simulink model "simulation_environment_thesis"  (R2024b)
settings: Solver=VariableStepAuto  StartTime=0.0  StopTime=3200  RelTol=1e-3
values from neighbouring files (symbols this model uses):
  A = [1, dt; 0, 1]    [estimate_and_control_fully_actuated_with_current.m]
  A1 = [1, 0, 0, 0, dt, 0 ... (first row only)    [estimate_and_control_fully_actuated_with_current.m]
  alpha = 10    [estimate_and_control_fully_actuated_with_current.m]
  alpha_xy_1 = 1    [estimate_and_control_fully_actuated_with_current.m]
  alpha_z_1 = 5    [estimate_and_control_fully_actuated_with_current.m]
  Az1 = [1, dt ... (first row only)    [estimate_and_control_fully_actuated_with_current.m]
  B = [dt; 0]    [estimate_and_control_fully_actuated_with_current.m]
  B1 = [dt, 0 ... (first row only)    [estimate_and_control_fully_actuated_with_current.m]
  Bz1 = [dt ... (first row only)    [estimate_and_control_fully_actuated_with_current.m]
  C1 = [1, 0, 0, 0, 0, 0 ... (first row only)    [estimate_and_control_fully_actuated_with_current.m]
  circumference_x = min_dist_from_ds*cos(theta)    [estimate_and_control_fully_actuated_with_current.m]
  circumference_y = min_dist_from_ds*sin(theta)    [estimate_and_control_fully_actuated_with_current.m]
  const_current = [-r; -s; 0; 0]    [estimate_and_control_fully_actuated_with_current.m]
  csi_psi_1 = 0.0003    [estimate_and_control_fully_actuated_with_current.m]
  csi_r_1 = 0.0003    [estimate_and_control_fully_actuated_with_current.m]
  csi_vcx_1 = 0.0003    [estimate_and_control_fully_actuated_with_current.m]
  csi_vcy_1 = 0.0003    [estimate_and_control_fully_actuated_with_current.m]
  csi_vcz_1 = 0.005    [estimate_and_control_fully_actuated_with_current.m]
  csi_x_1 = 0.003    [estimate_and_control_fully_actuated_with_current.m]
  csi_y_1 = 0.003    [estimate_and_control_fully_actuated_with_current.m]
  csi_z_1 = 0.005    [estimate_and_control_fully_actuated_with_current.m]
  Cz1 = [1, 0]    [estimate_and_control_fully_actuated_with_current.m]
  d = 1    [estimate_and_control_fully_actuated_with_current.m]
  delta_FOV = pi/7    (= 0.448798950513)    [estimate_and_control_fully_actuated_with_current.m]
  desired_speed = speed_0    [estimate_and_control_fully_actuated_with_current.m]
  dt = 1/Tsim    (= 0.005)    [estimate_and_control_fully_actuated_with_current.m]
  eta1 = deg2rad(0.5)    [estimate_and_control_fully_actuated_with_current.m]
  eta2 = deg2rad(0.5)    [estimate_and_control_fully_actuated_with_current.m]
  eta_psi_1 = 0.003    [estimate_and_control_fully_actuated_with_current.m]
  eta_x_1 = 0.03    [estimate_and_control_fully_actuated_with_current.m]
  eta_y_1 = 0.03    [estimate_and_control_fully_actuated_with_current.m]
  eta_z_1 = 0.005    [estimate_and_control_fully_actuated_with_current.m]
  G = dt*eye(2)    [estimate_and_control_fully_actuated_with_current.m]
  G1 = dt*eye(6)    [estimate_and_control_fully_actuated_with_current.m]
  Gz1 = dt*eye(2)    [estimate_and_control_fully_actuated_with_current.m]
  left_reference = L_x*[cos(NED_psi_ds); sin(NED_psi_ds); 0] + L_y_l*[-sin(NED_psi_ds); cos(NED_psi_ds); 0]    [estimate_and_control_fully_actuated_with_current.m]
  max_dist_from_ship = 60    [estimate_and_control_fully_actuated_with_current.m]
  min_dist_from_ds = 45    [estimate_and_control_fully_actuated_with_current.m]
  mu = -5    [estimate_and_control_fully_actuated_with_current.m]
  NED_psi_ds = -pi/2    (= -1.57079632679)    [estimate_and_control_fully_actuated_with_current.m]
  p_b1 = [-90; 90; 5]    [estimate_and_control_fully_actuated_with_current.m]
  position_t_0 = [0; 0; 0; 0]    [estimate_and_control_fully_actuated_with_current.m]
  psi_P = linspace(0,2*pi,360)'    [estimate_and_control_fully_actuated_with_current.m]
  Q = [csi1_kf^2, 0 ... (first row only)    [estimate_and_control_fully_actuated_with_current.m]
  Q1 = [csi_x_1_kf^2, 0, 0, 0, 0, 0 ... (first row only)    [estimate_and_control_fully_actuated_with_current.m]
  Qz1 = [csi_z_1_kf^2, 0 ... (first row only)    [estimate_and_control_fully_actuated_with_current.m]
  R = [eta1_kf^2, 0 ... (first row only)    [estimate_and_control_fully_actuated_with_current.m]
  R1 = [eta_x_1_kf^2, 0, 0 ... (first row only)    [estimate_and_control_fully_actuated_with_current.m]
  right_reference = L_x*[cos(NED_psi_ds); sin(NED_psi_ds); 0] + L_y_r*[sin(NED_psi_ds); -cos(NED_psi_ds); 0]    [estimate_and_control_fully_actuated_with_current.m]
  Rz1 = eta_z_1_kf^2    (= 1e-06)    [estimate_and_control_fully_actuated_with_current.m]
  speed_0 = [0.1, 0.1, 0, 0]'    [estimate_and_control_fully_actuated_with_current.m]
  tau_heave_sat = 30    [estimate_and_control_fully_actuated_with_current.m]
  tau_surge_sat = 10    [estimate_and_control_fully_actuated_with_current.m]
  tau_sway_sat = 30    [estimate_and_control_fully_actuated_with_current.m]
  tau_yaw_sat = 0.1    [estimate_and_control_fully_actuated_with_current.m]
  var_vm_x = 0.001    [estimate_and_control_fully_actuated_with_current.m]
  var_vm_y = 0.001    [estimate_and_control_fully_actuated_with_current.m]
  var_vm_z = 0.001    [estimate_and_control_fully_actuated_with_current.m]
undefined symbols (not defined in the file or in its folder): A2, alpha2, B2, csi1_2, csi2_2, csi3_2, csi4_2, csi5_2, csi6_2, csi7_2, csi8_2, eta1_2, eta2_2, eta3_2, eta4_2, eta5_2, eta6_2, G2, Q2, R2
libraries: simulink x60, simulink_extras x5
(part 1 of 16)
settings Model: Creator=ambra  LastModifiedBy=ambra

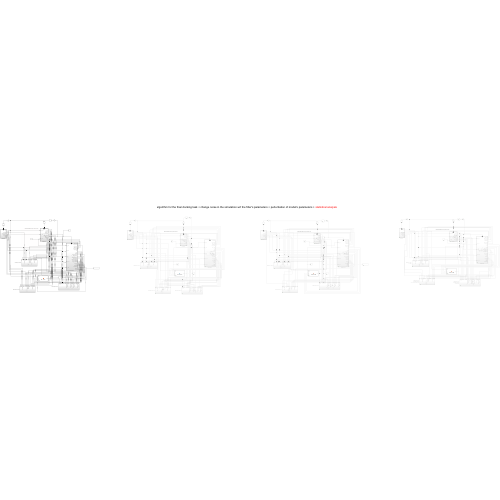
[thumbnail: Simulink's stored preview of the top-level diagram]
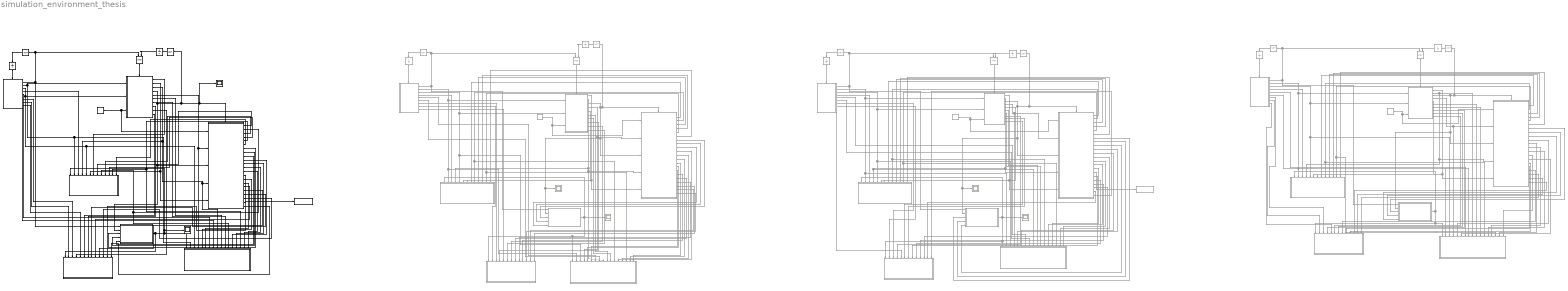
== system simulation_environment_thesis ==
  note: before transformation from ship coord to ds coord
  note: algorithm for the final docking task + change noise in the simulation wrt the filter's parameters + perturbation of model's parameters + statistical analysis
  note: before transformation from ship coord to ds coord
  note: before transformation from ship coord to ds coord
  note: before transformation from ship coord to ds coord
  AND21 : Logic  Commented=on  IconShape=distinctive  AllPortsSameDT=off  OutDataTypeStr=boolean
  AND22 : Logic  Commented=on  IconShape=distinctive  AllPortsSameDT=off  OutDataTypeStr=boolean
  AND23 : Logic  Commented=on  IconShape=distinctive  AllPortsSameDT=off  OutDataTypeStr=boolean
  AND24 : Logic  IconShape=distinctive  AllPortsSameDT=off  OutDataTypeStr=boolean
  Constant30 : Constant  Value=p_b1
  Constant31 : Constant  Commented=on  Value=p_b1
  Constant32 : Constant  Commented=on  Value=p_b1
  Constant33 : Constant  Commented=on  Value=p_b1
  MATLAB Function27 : MATLAB Function  TreatAsAtomicUnit=on
  MATLAB Function28 : MATLAB Function  Commented=on  TreatAsAtomicUnit=on
  MATLAB Function29 : MATLAB Function  Commented=on  TreatAsAtomicUnit=on
  MATLAB Function30 : MATLAB Function  Commented=on  TreatAsAtomicUnit=on
  NOT43 : Logic  Operator=NOT  IconShape=distinctive  AllPortsSameDT=off  OutDataTypeStr=boolean
  NOT44 : Logic  Operator=NOT  IconShape=distinctive  AllPortsSameDT=off  OutDataTypeStr=boolean
  NOT{45..50} (6 alike) : Logic  Commented=on  Operator=NOT  IconShape=distinctive  AllPortsSameDT=off  OutDataTypeStr=boolean
  "PHASE 1  " : Subsystem  Commented=on
  PHASE 1.21 : Subsystem  Commented=on
  PHASE 1.22 : Subsystem  Commented=on
  PHASE 1.24 : Subsystem
  "PHASE 2  " : Subsystem  Commented=on
  PHASE 2.21 : Subsystem  Commented=on
  PHASE 2.22 : Subsystem  Commented=on
  PHASE 2.24 : Subsystem
  "PHASE 3&4  " : Subsystem  Commented=on
  PHASE 3&4.21 : Subsystem  Commented=on
  PHASE 3&4.22 : Subsystem  Commented=on
  PHASE 3&4.24 : Subsystem
  Scope50 : Scope
  Scope51 : Scope
  Scope{53..54,56..57} (4 alike) : Scope  Commented=on
  To Workspace15 : ToWorkspace  VariableName=yaw_rel_error1  SampleTime=-1
  To Workspace16 : ToWorkspace  Commented=on  VariableName=yaw_rel_error  SampleTime=-1
  Unit Delay43 : UnitDelay  InitialCondition=1  SampleTime=-1  HasFrameUpgradeWarning=on
  Unit Delay44 : UnitDelay  InitialCondition=1  SampleTime=-1  HasFrameUpgradeWarning=on
  Unit Delay{45..50} (6 alike) : UnitDelay  Commented=on  InitialCondition=1  SampleTime=-1  HasFrameUpgradeWarning=on
  toWorkspace blocks for plotting : Subsystem  Commented=on
  toWorkspace blocks for statistical analysis on the error wrt the references : Subsystem  Commented=on
  "toWorkspace blocks for statistical analysis on the estimate  " : Subsystem  Commented=on
  toWorkspace blocks 21 : Subsystem  Commented=on
  toWorkspace blocks 22 : Subsystem  Commented=on
  toWorkspace blocks 24 : Subsystem
  toWorkspace blocks 25 : Subsystem
  toWorkspace blocks 26 : Subsystem  Commented=on
  toWorkspace blocks 27 : Subsystem  Commented=on
  toWorkspace blocks 29 : Subsystem
  toWorkspace blocks 30 : Subsystem  Commented=on
  toWorkspace blocks 31 : Subsystem  Commented=on
  wires:
  PHASE 1.21/3(p) -> toWorkspace blocks 26/3(p1), toWorkspace blocks 21/5(p1), PHASE 2.21/2(ship_p_auv)
  PHASE 1.21/1(stop_flag) -> NOT45/1, AND21/1, MATLAB Function28/1(stop1)
  Constant32/1 -> PHASE 3&4.22/1(ship_p_ds), PHASE 2.22/3(ship_p_ds)
  Constant31/1 -> PHASE 3&4.21/1(ship_p_ds), PHASE 2.21/3(ship_p_ds)
  PHASE 3&4.22/18(yaw_error) -> toWorkspace blocks 31/9(psi3)
  PHASE 2.24/2(s_p_auv) -> PHASE 3&4.24/5(ship_p_auv), toWorkspace blocks 24/6(p2)
  PHASE 3&4.21/16("z_err ") -> toWorkspace blocks 30/10(p3)
  PHASE 1.24/5(state_est_plane) -> toWorkspace blocks 25/7(state_est_plane_1)
  "PHASE 2  "/8(angle) -> toWorkspace blocks for statistical analysis on the error wrt the references/4(psi2)
  PHASE 1.24/6(state_est_z) -> toWorkspace blocks 25/8(state_est_z_1)
  "PHASE 2  "/9(error speed) -> toWorkspace blocks for statistical analysis on the error wrt the references/7(speed body2)
  "PHASE 3&4  "/13(state_est_plane3) -> "toWorkspace blocks for statistical analysis on the estimate  "/13(state_est_plane_3)
  "PHASE 3&4  "/11(state_est_plane4) -> "toWorkspace blocks for statistical analysis on the estimate  "/16(state_est_plane_4)
  Unit Delay48/1 -> AND22/2
  PHASE 3&4.24/21(angle 4) -> toWorkspace blocks 29/12(psi4)
  "PHASE 3&4  "/8(out1) -> MATLAB Function30/4(out1)
  PHASE 3&4.24/19(error speed) -> toWorkspace blocks 29/8(speed body3)
  PHASE 3&4.24/16(ds_p_auv_phase4) -> toWorkspace blocks 25/6(p4)
  "PHASE 2  "/10(z_ err) -> toWorkspace blocks for statistical analysis on the error wrt the references/6(p2)
  PHASE 3&4.21/4(speed body1) -> toWorkspace blocks 21/11(speed_body4)
  "PHASE 3&4  "/12(state_est_z3) -> "toWorkspace blocks for statistical analysis on the estimate  "/14(state_est_z_3)
  PHASE 3&4.24/6(ship_p_auv2) -> toWorkspace blocks 24/13(p4)
  PHASE 3&4.22/4(speed body1) -> toWorkspace blocks 22/11(speed_body4)
  "PHASE 2  "/4(ds_p_auv) -> "toWorkspace blocks for statistical analysis on the estimate  "/4(p2), "PHASE 3&4  "/2(ds_p_auv)
  "PHASE 3&4  "/17(yaw_error) -> toWorkspace blocks for statistical analysis on the error wrt the references/9(psi3)
  "PHASE 1  "/2(psi) -> "toWorkspace blocks for statistical analysis on the estimate  "/2(psi1), toWorkspace blocks for plotting/2(psi1), "PHASE 2  "/1(ship_psi_auv)
  "PHASE 1  "/3(p) -> "toWorkspace blocks for statistical analysis on the estimate  "/3(p1), toWorkspace blocks for plotting/5(p1), "PHASE 2  "/2(ship_p_auv)
  PHASE 3&4.24/18("z_err ") -> toWorkspace blocks 29/10(p3)
  Unit Delay43/1 -> PHASE 1.24/Enable
  PHASE 2.21/6(state_est_plane) -> toWorkspace blocks 26/10(state_est_plane_2)
  PHASE 3&4.21/17(error speed) -> toWorkspace blocks 30/8(speed body3)
  PHASE 2.24/6(state_est_plane) -> toWorkspace blocks 25/10(state_est_plane_2)
  NOT44/1 -> Unit Delay44/1
  "PHASE 3&4  "/10(ds_p_auv_3) -> "toWorkspace blocks for statistical analysis on the estimate  "/5(p3)
  PHASE 1.22/8(angle) -> toWorkspace blocks 31/2(psi1)
  PHASE 3&4.21/10(state_est_plane_3) -> toWorkspace blocks 26/13(state_est_plane_3)
  PHASE 3&4.21/6(ship_p_auv2) -> toWorkspace blocks 21/13(p4)
  PHASE 2.22/9(error speed) -> toWorkspace blocks 31/7(speed body2)
  "PHASE 1  "/9(z_ err) -> toWorkspace blocks for statistical analysis on the error wrt the references/5(p1)
  Unit Delay49/1 -> AND23/2
  Unit Delay44/1 -> AND24/2
  "PHASE 1  "/6(state_est_z) -> "toWorkspace blocks for statistical analysis on the estimate  "/8(state_est_z_1)
  "PHASE 3&4  "/7(out) -> MATLAB Function30/3(out)
  PHASE 3&4.22/12(state_est_plane4) -> toWorkspace blocks 27/16(state_est_plane_4)
  "PHASE 3&4  "/19(z_ err 4) -> toWorkspace blocks for statistical analysis on the error wrt the references/13(p4)
  PHASE 1.21/9(z_ err) -> toWorkspace blocks 30/5(p1)
  PHASE 3&4.24/15(ds_p_auv_phase3) -> toWorkspace blocks 25/5(p3)
  "PHASE 2  "/2(s_psi_auv) -> toWorkspace blocks for plotting/4(psi2), "PHASE 3&4  "/4(ship_psi_auv), "toWorkspace blocks for statistical analysis on the estimate  "/9(psi2)
  PHASE 1.22/6(state_z) -> toWorkspace blocks 27/8(state_est_z_1)
  PHASE 3&4.22/21(angle 4) -> toWorkspace blocks 31/12(psi4)
  PHASE 1.21/6(state_est_z) -> toWorkspace blocks 26/8(state_est_z_1)
  PHASE 1.24/2(psi) -> PHASE 2.24/1(ship_psi_auv), toWorkspace blocks 25/2(psi1), toWorkspace blocks 24/2(psi1)
  PHASE 3&4.22/10(ds_p_auv_3) -> toWorkspace blocks 27/5(p3)
  AND24/1 -> PHASE 2.24/Enable
  PHASE 3&4.22/20(error speed 4) -> toWorkspace blocks 31/11(speed_body4)
  PHASE 1.21/8(angle) -> toWorkspace blocks 30/2(psi1)
  PHASE 3&4.22/1(speed body) -> toWorkspace blocks 22/8(speed body3)
  PHASE 3&4.24/22(z err 4) -> toWorkspace blocks 29/13(p4)
  PHASE 3&4.24/1(speed body) -> toWorkspace blocks 24/8(speed body3)
  "PHASE 1  "/5(state_est_plane) -> "toWorkspace blocks for statistical analysis on the estimate  "/7(state_est_plane_1)
  "PHASE 1  "/7(speed error) -> toWorkspace blocks for statistical analysis on the error wrt the references/3(body_speed_1)
  PHASE 3&4.24/5(ship_psi_auv2) -> toWorkspace blocks 24/12(psi4), toWorkspace blocks 25/15(psi4)
  Unit Delay46/1 -> AND21/2
  MATLAB Function27/1(state) -> toWorkspace blocks 24/1(state), Scope50/1, toWorkspace blocks 25/1(state), toWorkspace blocks 29/1(state)
  PHASE 3&4.22/22("z_ err 4 ") -> toWorkspace blocks 31/13(p4)
  PHASE 3&4.22/8(out1) -> MATLAB Function29/4(out1)
  PHASE 3&4.21/20(angle 4) -> toWorkspace blocks 30/12(psi4)
  PHASE 2.22/10(z_ err) -> toWorkspace blocks 31/6(p2)
  PHASE 1.21/7(error speed) -> toWorkspace blocks 30/3(body_speed_1)
  "PHASE 2  "/3(stop_flag_2) -> "PHASE 3&4  "/3(stop_flag), MATLAB Function30/2(stop2), "PHASE 3&4  "/Enable, NOT49/1
  "PHASE 1  "/1(stop_flag) -> AND23/1, NOT50/1, MATLAB Function30/1(stop1)
  PHASE 2.22/2(s_psi_auv) -> PHASE 3&4.22/4(ship_psi_auv), toWorkspace blocks 27/9(psi2), toWorkspace blocks 22/4(psi2)
  PHASE 3&4.21/8(out1) -> MATLAB Function28/4(out1)
  PHASE 3&4.24/3(ship_p_auv1) -> toWorkspace blocks 24/10(p3)
  PHASE 3&4.22/16(yaw_rel_err) -> To Workspace16/1
  PHASE 1.22/1(stop_flag) -> NOT47/1, AND22/1, MATLAB Function29/1(stop1)
  PHASE 3&4.22/19(z_ err) -> toWorkspace blocks 31/10(p3)
  PHASE 1.21/4(body speed) -> toWorkspace blocks 21/3(body_speed_1)
  PHASE 2.21/8(angle) -> toWorkspace blocks 30/4(psi2)
  MATLAB Function29/1(state) -> toWorkspace blocks 31/1(state), toWorkspace blocks 27/1(state), Scope56/1, toWorkspace blocks 22/1(state)
  PHASE 3&4.24/10(state_est_plane_3) -> toWorkspace blocks 25/13(state_est_plane_3)
  PHASE 2.24/5(ds_p_auv) -> toWorkspace blocks 25/4(p2), PHASE 3&4.24/2(ds_p_auv)
  PHASE 2.21/9(error speed) -> toWorkspace blocks 30/7(speed body2)
  PHASE 1.24/7(error speed) -> toWorkspace blocks 29/3(body_speed_1)
  PHASE 3&4.24/7(out) -> MATLAB Function27/3(out)
  PHASE 3&4.21/14(ds_p_auv_3) -> toWorkspace blocks 26/5(p3)
  PHASE 2.22/3(stop_flag_2) -> PHASE 3&4.22/3(stop_flag), Scope57/1, MATLAB Function29/2(stop2), NOT48/1, PHASE 3&4.22/Enable
  PHASE 1.22/7(error speed) -> toWorkspace blocks 31/3(body_speed_1)
  PHASE 3&4.24/14(state_est_yaw_rel_4) -> To Workspace15/1
  PHASE 3&4.22/17(error speed) -> toWorkspace blocks 31/8(speed body3)
  PHASE 1.22/3(p) -> toWorkspace blocks 27/3(p1), toWorkspace blocks 22/5(p1), PHASE 2.22/2(ship_p_auv)
  PHASE 3&4.22/11(ds_p_auv4) -> toWorkspace blocks 27/6(p4)
  NOT45/1 -> Unit Delay45/1
  PHASE 2.21/1(s_p_auv) -> PHASE 3&4.21/5(ship_p_auv), toWorkspace blocks 21/6(p2)
  PHASE 2.22/6(state_est_plane) -> toWorkspace blocks 27/10(state_est_plane_2)
  PHASE 3&4.21/3(ship_p_auv1) -> toWorkspace blocks 21/10(p3)
  PHASE 2.21/7(state_est_z) -> toWorkspace blocks 26/11(state_est_z_2)
  PHASE 3&4.24/17(yaw_error) -> toWorkspace blocks 29/9(psi3)
  PHASE 2.24/7(state_est_z) -> toWorkspace blocks 25/11(state_est_z_2)
  Unit Delay50/1 -> "PHASE 1  "/Enable
  PHASE 3&4.22/6(ship_p_auv2) -> toWorkspace blocks 22/13(p4)
  PHASE 1.21/5(state_est_plane) -> toWorkspace blocks 26/7(state_est_plane_1)
  PHASE 3&4.24/4(speed body1) -> toWorkspace blocks 24/11(speed_body4)
  "PHASE 3&4  "/18(error speed) -> toWorkspace blocks for statistical analysis on the error wrt the references/8(speed body3)
  PHASE 2.21/2(s_psi_auv) -> PHASE 3&4.21/4(ship_psi_auv), toWorkspace blocks 26/9(psi2), toWorkspace blocks 21/4(psi2)
  PHASE 3&4.24/20(error speed 4) -> toWorkspace blocks 29/11(speed_body4)
  PHASE 2.22/4(ds_p_auv) -> toWorkspace blocks 27/4(p2), PHASE 3&4.22/2(ds_p_auv)
  PHASE 3&4.22/9(docked) -> MATLAB Function29/5(docked)
  PHASE 3&4.21/11(state_est_z_3) -> toWorkspace blocks 26/14(state_est_z_3)
  PHASE 2.24/3(s_psi_auv) -> toWorkspace blocks 25/9(psi2), PHASE 3&4.24/4(ship_psi_auv), toWorkspace blocks 24/4(psi2)
  AND22/1 -> PHASE 2.22/Enable
  PHASE 1.24/1(stop_flag) -> AND24/1, NOT43/1, MATLAB Function27/1(stop1)
  PHASE 2.24/10("z_err ") -> toWorkspace blocks 29/6(p2)
  PHASE 1.22/4(body speed) -> toWorkspace blocks 22/3(body_speed_1)
  PHASE 3&4.22/15(state_est_z3) -> toWorkspace blocks 27/14(state_est_z_3)
  "PHASE 1  "/4(body speed) -> toWorkspace blocks for plotting/3(body_speed_1)
  PHASE 1.24/8(angle) -> toWorkspace blocks 29/2(psi1)
  PHASE 2.24/9(angle) -> toWorkspace blocks 29/4(psi2)
  MATLAB Function30/1(state) -> toWorkspace blocks for statistical analysis on the error wrt the references/1(state), "toWorkspace blocks for statistical analysis on the estimate  "/1(state), toWorkspace blocks for plotting/1(state)
  "PHASE 3&4  "/15(ds_p_auv4) -> "toWorkspace blocks for statistical analysis on the estimate  "/6(p4)
  PHASE 3&4.24/2(ship_psi_auv1) -> toWorkspace blocks 24/9(psi3), toWorkspace blocks 25/12(psi3)
  "PHASE 3&4  "/5(ship_psi_auv2) -> "toWorkspace blocks for statistical analysis on the estimate  "/15(psi4), toWorkspace blocks for plotting/12(psi4)
  PHASE 1.22/9("z_err ") -> toWorkspace blocks 31/5(p1)
  "PHASE 3&4  "/3(ship_p_auv1) -> toWorkspace blocks for plotting/10(p3)
  PHASE 1.24/4(body speed) -> toWorkspace blocks 24/3(body_speed_1)
  "PHASE 2  "/6(state_est_plane) -> "toWorkspace blocks for statistical analysis on the estimate  "/10(state_est_plane_2)
  PHASE 1.22/2(psi) -> toWorkspace blocks 27/2(psi1), toWorkspace blocks 22/2(psi1), PHASE 2.22/1(ship_psi_auv)
  PHASE 2.22/8(angle) -> toWorkspace blocks 31/4(psi2)
  PHASE 3&4.22/13(state_est_z4) -> toWorkspace blocks 27/17(state_est_z_4)
  PHASE 3&4.21/2(ship_psi_auv1) -> toWorkspace blocks 26/12(psi3), toWorkspace blocks 21/9(psi3)
  PHASE 2.22/5(body speed) -> toWorkspace blocks 22/7(speed body2)
  PHASE 1.22/5(state_plane) -> toWorkspace blocks 27/7(state_est_plane_1)
  PHASE 3&4.22/2(ship_psi_auv1) -> toWorkspace blocks 27/12(psi3), toWorkspace blocks 22/9(psi3)
  PHASE 3&4.22/7(out) -> MATLAB Function29/3(out)
  NOT43/1 -> Unit Delay43/1
  "PHASE 3&4  "/16(z_ err) -> toWorkspace blocks for statistical analysis on the error wrt the references/10(p3)
  PHASE 3&4.21/9(docked) -> MATLAB Function28/5(docked)
  PHASE 3&4.21/5(ship_psi_auv2) -> toWorkspace blocks 26/15(psi4), toWorkspace blocks 21/12(psi4)
  "PHASE 3&4  "/21(error speed 4) -> toWorkspace blocks for statistical analysis on the error wrt the references/11(speed_body4)
  PHASE 3&4.21/12(state_est_plane_4) -> toWorkspace blocks 26/16(state_est_plane_4)
  PHASE 1.24/3(p) -> toWorkspace blocks 25/3(p1), toWorkspace blocks 24/5(p1), PHASE 2.24/2(ship_p_auv)
  PHASE 3&4.24/12(state_est_plane_4) -> toWorkspace blocks 25/16(state_est_plane_4)
  PHASE 3&4.21/21(error speed 4) -> toWorkspace blocks 30/11(speed_body4)
  PHASE 3&4.21/18(yaw_error) -> toWorkspace blocks 30/9(psi3)
  AND23/1 -> "PHASE 2  "/Enable
  MATLAB Function28/1(state) -> toWorkspace blocks 30/1(state), toWorkspace blocks 26/1(state), toWorkspace blocks 21/1(state), Scope53/1
  PHASE 3&4.21/1(speed body) -> toWorkspace blocks 21/8(speed body3)
  "PHASE 3&4  "/6(ship_p_auv2) -> toWorkspace blocks for plotting/13(p4)
  "PHASE 1  "/8(yaw_error) -> toWorkspace blocks for statistical analysis on the error wrt the references/2(psi1)
  "PHASE 2  "/5(body speed) -> toWorkspace blocks for plotting/7(speed body2)
  "PHASE 3&4  "/1(speed body) -> toWorkspace blocks for plotting/8(speed body3)
  PHASE 2.21/4(ds_p_auv) -> toWorkspace blocks 26/4(p2), PHASE 3&4.21/2(ds_p_auv)
  Constant30/1 -> PHASE 3&4.24/1(ship_p_ds), PHASE 2.24/3(ship_p_ds)
  Unit Delay45/1 -> PHASE 1.21/Enable
  Unit Delay47/1 -> PHASE 1.22/Enable
  PHASE 2.22/7(state_est_z) -> toWorkspace blocks 27/11(state_est_z_2)
  "PHASE 3&4  "/9(docked) -> MATLAB Function30/5(docked)
  PHASE 3&4.24/8(out1) -> MATLAB Function27/4(out1)
  PHASE 3&4.24/13(state_est_z_4) -> toWorkspace blocks 25/17(state_est_z_4)
  "PHASE 3&4  "/14(state_est_z4) -> "toWorkspace blocks for statistical analysis on the estimate  "/17(state_est_z_4)
  PHASE 3&4.21/7(out) -> MATLAB Function28/3(out)
  NOT50/1 -> Unit Delay50/1
  PHASE 3&4.21/13(state_est_z_4) -> toWorkspace blocks 26/17(state_est_z_4)
  PHASE 3&4.22/5(ship_psi_auv2) -> toWorkspace blocks 27/15(psi4), toWorkspace blocks 22/12(psi4)
  PHASE 1.21/2(psi) -> toWorkspace blocks 26/2(psi1), toWorkspace blocks 21/2(psi1), PHASE 2.21/1(ship_psi_auv)
  NOT49/1 -> Unit Delay49/1
  PHASE 3&4.22/14(state_est_plane3) -> toWorkspace blocks 27/13(state_est_plane_3)
  Constant33/1 -> "PHASE 3&4  "/1(ship_p_ds), "PHASE 2  "/3(ship_p_ds)
  PHASE 3&4.24/9(docked) -> MATLAB Function27/5(docked)
  PHASE 2.21/5(body speed) -> toWorkspace blocks 21/7(speed body2)
  PHASE 2.21/10(z_ err) -> toWorkspace blocks 30/6(p2)
  "PHASE 3&4  "/2(ship_psi_auv1) -> "toWorkspace blocks for statistical analysis on the estimate  "/12(psi3), toWorkspace blocks for plotting/9(psi3)
  "PHASE 2  "/1(s_p_auv) -> "PHASE 3&4  "/5(ship_p_auv), toWorkspace blocks for plotting/6(p2)
  PHASE 3&4.21/15(ds_p_auv_4) -> toWorkspace blocks 26/6(p4)
  PHASE 2.22/1(s_p_auv) -> PHASE 3&4.22/5(ship_p_auv), toWorkspace blocks 22/6(p2)
  PHASE 2.24/8(error speed) -> toWorkspace blocks 29/7(speed body2)
  PHASE 2.21/3(stop_flag_2) -> PHASE 3&4.21/3(stop_flag), Scope54/1, MATLAB Function28/2(stop2), PHASE 3&4.21/Enable, NOT46/1
  PHASE 2.24/1(speed body) -> toWorkspace blocks 24/7(speed body2)
  "PHASE 2  "/7(state_est_z) -> "toWorkspace blocks for statistical analysis on the estimate  "/11(state_est_z_2)
  PHASE 3&4.22/3(ship_p_auv1) -> toWorkspace blocks 22/10(p3)
  NOT46/1 -> Unit Delay46/1
  "PHASE 3&4  "/20(angle 4) -> toWorkspace blocks for statistical analysis on the error wrt the references/12(psi4)
  NOT47/1 -> Unit Delay47/1
  PHASE 3&4.21/19(z_err 4) -> toWorkspace blocks 30/13(p4)
  AND21/1 -> PHASE 2.21/Enable
  PHASE 2.24/4(stop_flag_2) -> NOT44/1, PHASE 3&4.24/Enable, Scope51/1, MATLAB Function27/2(stop2), PHASE 3&4.24/3(stop_flag)
  PHASE 3&4.24/11(state_est_z_3) -> toWorkspace blocks 25/14(state_est_z_3)
  "PHASE 3&4  "/4(speed body1) -> toWorkspace blocks for plotting/11(speed_body4)
  PHASE 1.24/9("z_err ") -> toWorkspace blocks 29/5(p1)
  NOT48/1 -> Unit Delay48/1

== system simulation_environment_thesis/PHASE 1   ==
  note: PHASE 1
  Enable : EnablePort
  CONTROLLER1 : Subsystem
  Constant2 : Constant  Value=p_b1(3)
  Data Store Write : DataStoreWrite  DataStoreName=x_hat_12_xy_4  IndexOptions=Index vector (dialog)  Indices=1  OutputSizes=1
  Data Store Write1 : DataStoreWrite  DataStoreName=x_hat_12_z_4  IndexOptions=Index vector (dialog)  Indices=1  OutputSizes=1
  Data Store Write2 : DataStoreWrite  DataStoreName=P0_12_xy_4  IndexOptions=Index vector (dialog)  Indices=1  OutputSizes=1
  Data Store Write3 : DataStoreWrite  DataStoreName=P0_12_z_4  IndexOptions=Index vector (dialog)  Indices=1  OutputSizes=1
  REFERENCE GENERATOR : Subsystem
  Scope : Scope
  Selector : Selector  InputPortWidth=4  IndexOptions=Index vector (dialog)  Indices=[1 2]  OutputSizes=1
  Sum : Sum  Inputs=|+-
  Sum1 : Sum  Inputs=|-+
  TAU : Scope
  dynamic + kinematic models1 : Subsystem
  v error (robot frame) : Scope
  "x,y,psi,r,vc estimate, XY PLANE from x,y,psi" : Subsystem
  "z, v_c_z estimate from DEPTH measurement" : Subsystem
  z_err : Scope
  stop_flag : Outport
  psi : Outport  Port=2
  p : Outport  Port=3
  body speed : Outport  Port=4
  state_est_plane : Outport  Port=5
  state_est_z : Outport  Port=6
  speed error : Outport  Port=7
  yaw_error : Outport  Port=8
  z_ err : Outport  Port=9
  wires:
  dynamic + kinematic models1/2(v_tot inert) -> Selector/1
  CONTROLLER1/1(tau) -> dynamic + kinematic models1/1(tau), TAU/1
  dynamic + kinematic models1/3(psi) -> "x,y,psi,r,vc estimate, XY PLANE from x,y,psi"/3(psi), psi/1, dynamic + kinematic models1/3(yaw prev)
  Selector/1 -> "x,y,psi,r,vc estimate, XY PLANE from x,y,psi"/2(inert_vel)
  dynamic + kinematic models1/1(error speed) -> CONTROLLER1/1(v_err), v error (robot frame)/1, speed error/1
  dynamic + kinematic models1/4(p) -> "z, v_c_z estimate from DEPTH measurement"/1(p), "x,y,psi,r,vc estimate, XY PLANE from x,y,psi"/1(p), p/1
  "z, v_c_z estimate from DEPTH measurement"/1(z_est) -> Sum/2
  Constant2/1 -> Sum/1
  Sum/1 -> z_ err/1, z_err/1, CONTROLLER1/4(z_err)
  "x,y,psi,r,vc estimate, XY PLANE from x,y,psi"/2(psi_est) -> Sum1/1
  Sum1/1 -> Scope/1, CONTROLLER1/3(yaw_err), yaw_error/1
  dynamic + kinematic models1/5(body speed) -> body speed/1
  "x,y,psi,r,vc estimate, XY PLANE from x,y,psi"/4(state_est) -> Data Store Write/1
  "z, v_c_z estimate from DEPTH measurement"/2(state_est) -> Data Store Write1/1
  "x,y,psi,r,vc estimate, XY PLANE from x,y,psi"/5(P_knext_knext) -> Data Store Write2/1
  "z, v_c_z estimate from DEPTH measurement"/3(P_k_k) -> Data Store Write3/1
  "x,y,psi,r,vc estimate, XY PLANE from x,y,psi"/6(state_est_plane) -> state_est_plane/1
  "z, v_c_z estimate from DEPTH measurement"/4(state_est_z) -> state_est_z/1
  REFERENCE GENERATOR/1(speed) -> dynamic + kinematic models1/2(ref speed), CONTROLLER1/2(speed_0)
  "x,y,psi,r,vc estimate, XY PLANE from x,y,psi"/1(xy_est) -> REFERENCE GENERATOR/1(p)
  "x,y,psi,r,vc estimate, XY PLANE from x,y,psi"/3(v_c_xy) -> REFERENCE GENERATOR/2(v_c)
  REFERENCE GENERATOR/2(yaw_dock) -> Sum1/2
  REFERENCE GENERATOR/3(stop_flag) -> stop_flag/1

== system simulation_environment_thesis/PHASE 1.21 ==
  note: USO I VALORI NOMINALI, NON HO NESSUN FEEDBACK SULLE VELOCITà EFFETTIVE NEL BODY FRAME
  note: ship reference frame
  note: PHASE 1:
  Enable : EnablePort
  CONTROLLER1 : Subsystem
  Constant : Constant  Value=p_b1
  Constant1 : Constant  Value=max_dist_from_ship
  Constant2 : Constant  Value=p_b1(3)
  Constant5 : Constant  Value=desired_speed
  Data Store Write : DataStoreWrite  DataStoreName=x_hat_12_xy_2  IndexOptions=Index vector (dialog)  Indices=1  OutputSizes=1
  Data Store Write1 : DataStoreWrite  DataStoreName=x_hat_12_z_2  IndexOptions=Index vector (dialog)  Indices=1  OutputSizes=1
  Data Store Write2 : DataStoreWrite  DataStoreName=P0_12_xy_2  IndexOptions=Index vector (dialog)  Indices=1  OutputSizes=1
  Data Store Write3 : DataStoreWrite  DataStoreName=P0_12_z_2  IndexOptions=Index vector (dialog)  Indices=1  OutputSizes=1
  MATLAB Function : MATLAB Function  TreatAsAtomicUnit=on
  MATLAB Function7 : MATLAB Function  TreatAsAtomicUnit=on
  Memory : Memory
  Scope : Scope
  Selector : Selector  InputPortWidth=4  IndexOptions=Index vector (dialog)  Indices=[1 2]  OutputSizes=1
  Sum : Sum  Inputs=|+-
  Sum1 : Sum  Inputs=|-+
  TAU1 : Scope
  dynamic + kinematic models3 : Subsystem
  v error (robot frame)4 : Scope
  "x,y,psi,r,vc estimate, XY PLANE from x,y,psi" : Subsystem
  "z, v_c_z estimate from DEPTH measurement" : Subsystem
  z_err : Scope
  stop_flag : Outport
  psi : Outport  Port=2
  p : Outport  Port=3
  body speed : Outport  Port=4
  state_est_plane : Outport  Port=5
  state_est_z : Outport  Port=6
  error speed : Outport  Port=7
  angle : Outport  Port=8
  z_ err : Outport  Port=9
  wires:
  dynamic + kinematic models3/2(v_tot inert) -> Selector/1
  CONTROLLER1/1(tau) -> dynamic + kinematic models3/1(tau), TAU1/1
  dynamic + kinematic models3/3(psi) -> dynamic + kinematic models3/3(yaw prev), "x,y,psi,r,vc estimate, XY PLANE from x,y,psi"/3(psi), psi/1
  Constant5/1 -> CONTROLLER1/2(speed_0), MATLAB Function7/5(speed), dynamic + kinematic models3/2(ref speed)
  Selector/1 -> "x,y,psi,r,vc estimate, XY PLANE from x,y,psi"/2(inert_vel)
  dynamic + kinematic models3/1(error speed) -> CONTROLLER1/1(v_err), error speed/1, v error (robot frame)4/1
  dynamic + kinematic models3/4(p) -> "z, v_c_z estimate from DEPTH measurement"/1(p), p/1, "x,y,psi,r,vc estimate, XY PLANE from x,y,psi"/1(p)
  "z, v_c_z estimate from DEPTH measurement"/1(z_est) -> Sum/2
  Constant2/1 -> Sum/1
  Sum/1 -> z_ err/1, z_err/1, CONTROLLER1/4(z_err)
  "x,y,psi,r,vc estimate, XY PLANE from x,y,psi"/1(xy_est) -> MATLAB Function7/3(p)
  "x,y,psi,r,vc estimate, XY PLANE from x,y,psi"/3(v_c_xy) -> MATLAB Function7/2(v_c)
  Constant/1 -> MATLAB Function7/4(beacon)
  Constant1/1 -> MATLAB Function7/6(max_distance_from_ship)
  Memory/1 -> MATLAB Function7/1("stop_flag ")
  MATLAB Function7/2(stop_flag) -> Memory/1, stop_flag/1
  "x,y,psi,r,vc estimate, XY PLANE from x,y,psi"/2(psi_est) -> Sum1/1
  MATLAB Function7/1(yaw_desired) -> Sum1/2
  Sum1/1 -> MATLAB Function/1("angle ")
  MATLAB Function/1(angle) -> angle/1, CONTROLLER1/3(yaw_err), Scope/1
  dynamic + kinematic models3/5(body speed) -> body speed/1
  "x,y,psi,r,vc estimate, XY PLANE from x,y,psi"/4(state_est) -> Data Store Write/1
  "z, v_c_z estimate from DEPTH measurement"/2(state_est) -> Data Store Write1/1
  "x,y,psi,r,vc estimate, XY PLANE from x,y,psi"/5(P_knext_knext) -> Data Store Write2/1
  "z, v_c_z estimate from DEPTH measurement"/3(P_k_k) -> Data Store Write3/1
  "x,y,psi,r,vc estimate, XY PLANE from x,y,psi"/6(state_est_plane) -> state_est_plane/1
  "z, v_c_z estimate from DEPTH measurement"/4(state_est_z) -> state_est_z/1

== system simulation_environment_thesis/PHASE 1.22 ==
  note: USO I VALORI NOMINALI, NON HO NESSUN FEEDBACK SULLE VELOCITà EFFETTIVE NEL BODY FRAME
  note: ship reference frame
  note: PHASE 1:
  Enable : EnablePort
  CONTROLLER1 : Subsystem
  Constant : Constant  Value=p_b1
  Constant1 : Constant  Value=max_dist_from_ship
  Constant2 : Constant  Value=p_b1(3)
  Constant5 : Constant  Value=desired_speed
  Data Store Write : DataStoreWrite  DataStoreName=x_hat_12_xy_3  IndexOptions=Index vector (dialog)  Indices=1  OutputSizes=1
  Data Store Write1 : DataStoreWrite  DataStoreName=x_hat_12_z_3  IndexOptions=Index vector (dialog)  Indices=1  OutputSizes=1
  Data Store Write2 : DataStoreWrite  DataStoreName=P0_12_xy_3  IndexOptions=Index vector (dialog)  Indices=1  OutputSizes=1
  Data Store Write3 : DataStoreWrite  DataStoreName=P0_12_z_3  IndexOptions=Index vector (dialog)  Indices=1  OutputSizes=1
  MATLAB Function : MATLAB Function  TreatAsAtomicUnit=on
  MATLAB Function7 : MATLAB Function  TreatAsAtomicUnit=on
  Memory : Memory
  Scope : Scope
  Selector : Selector  InputPortWidth=4  IndexOptions=Index vector (dialog)  Indices=[1 2]  OutputSizes=1
  Sum : Sum  Inputs=|+-
  Sum1 : Sum  Inputs=|-+
  TAU1 : Scope
  dynamic + kinematic models1 : Subsystem
  v error (robot frame)4 : Scope
  "x,y,psi,r,vc estimate, XY PLANE from x,y,psi" : Subsystem
  "z, v_c_z estimate from DEPTH measurement" : Subsystem
  z_err : Scope
  stop_flag : Outport
  psi : Outport  Port=2
  p : Outport  Port=3
  body speed : Outport  Port=4
  state_plane : Outport  Port=5
  state_z : Outport  Port=6
  error speed : Outport  Port=7
  angle : Outport  Port=8
  "z_err " : Outport  Port=9
  wires:
  dynamic + kinematic models1/2(v_tot inert) -> Selector/1
  CONTROLLER1/1(tau) -> dynamic + kinematic models1/1(tau), TAU1/1
  dynamic + kinematic models1/3(psi) -> dynamic + kinematic models1/3(yaw prev), "x,y,psi,r,vc estimate, XY PLANE from x,y,psi"/3(psi), psi/1
  Selector/1 -> "x,y,psi,r,vc estimate, XY PLANE from x,y,psi"/2(inert_vel)
  Constant5/1 -> CONTROLLER1/2(speed_0), dynamic + kinematic models1/2(ref speed), MATLAB Function7/5(speed)
  dynamic + kinematic models1/1(error speed) -> CONTROLLER1/1(v_err), v error (robot frame)4/1, error speed/1
  dynamic + kinematic models1/4(p) -> "z, v_c_z estimate from DEPTH measurement"/1(p), p/1, "x,y,psi,r,vc estimate, XY PLANE from x,y,psi"/1(p)
  "z, v_c_z estimate from DEPTH measurement"/1(z_est) -> Sum/2
  Constant2/1 -> Sum/1
  Sum/1 -> "z_err "/1, z_err/1, CONTROLLER1/4(z_err)
  "x,y,psi,r,vc estimate, XY PLANE from x,y,psi"/1(xy_est) -> MATLAB Function7/3(p)
  "x,y,psi,r,vc estimate, XY PLANE from x,y,psi"/3(v_c_xy) -> MATLAB Function7/2(v_c)
  Constant/1 -> MATLAB Function7/4(beacon)
  Constant1/1 -> MATLAB Function7/6(max_distance_from_ship)
  Memory/1 -> MATLAB Function7/1("stop_flag ")
  MATLAB Function7/2(stop_flag) -> Memory/1, stop_flag/1
  "x,y,psi,r,vc estimate, XY PLANE from x,y,psi"/2(psi_est) -> Sum1/1
  MATLAB Function7/1(yaw_desired) -> Sum1/2
  Sum1/1 -> MATLAB Function/1("angle ")
  MATLAB Function/1(angle) -> angle/1, CONTROLLER1/3(yaw_err), Scope/1
  dynamic + kinematic models1/5(body speed) -> body speed/1
  "x,y,psi,r,vc estimate, XY PLANE from x,y,psi"/4(state_est) -> Data Store Write/1
  "z, v_c_z estimate from DEPTH measurement"/2(state_est) -> Data Store Write1/1
  "x,y,psi,r,vc estimate, XY PLANE from x,y,psi"/5(P_knext_knext) -> Data Store Write2/1
  "z, v_c_z estimate from DEPTH measurement"/3(P_k_k) -> Data Store Write3/1
  "z, v_c_z estimate from DEPTH measurement"/4(state_z) -> state_z/1
  "x,y,psi,r,vc estimate, XY PLANE from x,y,psi"/6(state_plane) -> state_plane/1

== system simulation_environment_thesis/PHASE 1.24 ==
  note: USO I VALORI NOMINALI, NON HO NESSUN FEEDBACK SULLE VELOCITà EFFETTIVE NEL BODY FRAME
  note: ship reference frame
  note: PHASE 1:
  Enable : EnablePort
  CONTROLLER1 : Subsystem
  Constant : Constant  Value=p_b1
  Constant1 : Constant  Value=max_dist_from_ship
  Constant2 : Constant  Value=p_b1(3)
  Constant5 : Constant  Value=desired_speed
  Data Store Write : DataStoreWrite  DataStoreName=x_hat_12_xy_1  IndexOptions=Index vector (dialog)  Indices=1  OutputSizes=1
  Data Store Write1 : DataStoreWrite  DataStoreName=x_hat_12_z_1  IndexOptions=Index vector (dialog)  Indices=1  OutputSizes=1
  Data Store Write2 : DataStoreWrite  DataStoreName=P0_12_xy_1  IndexOptions=Index vector (dialog)  Indices=1  OutputSizes=1
  Data Store Write3 : DataStoreWrite  DataStoreName=P0_12_z_1  IndexOptions=Index vector (dialog)  Indices=1  OutputSizes=1
  MATLAB Function : MATLAB Function  TreatAsAtomicUnit=on
  MATLAB Function7 : MATLAB Function  TreatAsAtomicUnit=on
  Memory : Memory
  Scope : Scope
  Selector : Selector  InputPortWidth=4  IndexOptions=Index vector (dialog)  Indices=[1 2]  OutputSizes=1
  Sum : Sum  Inputs=|+-
  Sum1 : Sum  Inputs=|-+
  TAU1 : Scope
  dynamic + kinematic models1 : Subsystem
  v error (robot frame)4 : Scope
  "x,y,psi,r,vc estimate, XY PLANE from x,y,psi" : Subsystem
  "z, v_c_z estimate from DEPTH measurement" : Subsystem
  z_err : Scope
  stop_flag : Outport
  psi : Outport  Port=2
  p : Outport  Port=3
  body speed : Outport  Port=4
  state_est_plane : Outport  Port=5
  state_est_z : Outport  Port=6
  error speed : Outport  Port=7
  angle : Outport  Port=8
  "z_err " : Outport  Port=9
  wires:
  dynamic + kinematic models1/2(v_tot inert) -> Selector/1
  CONTROLLER1/1(tau) -> dynamic + kinematic models1/1(tau), TAU1/1
  dynamic + kinematic models1/3(psi) -> dynamic + kinematic models1/3(yaw prev), "x,y,psi,r,vc estimate, XY PLANE from x,y,psi"/3(psi), psi/1
  dynamic + kinematic models1/4(body speed) -> body speed/1
  Selector/1 -> "x,y,psi,r,vc estimate, XY PLANE from x,y,psi"/2(inert_vel)
  Constant5/1 -> CONTROLLER1/2(speed_0), dynamic + kinematic models1/2(ref speed), MATLAB Function7/5(speed)
  dynamic + kinematic models1/1(error speed) -> error speed/1, CONTROLLER1/1(v_err), v error (robot frame)4/1
  Constant2/1 -> Sum/1
  Sum/1 -> "z_err "/1, z_err/1, CONTROLLER1/4(z_err)
  "x,y,psi,r,vc estimate, XY PLANE from x,y,psi"/1(xy_est) -> MATLAB Function7/3(p)
  "x,y,psi,r,vc estimate, XY PLANE from x,y,psi"/3(v_c_xy) -> MATLAB Function7/2(v_c)
  Constant/1 -> MATLAB Function7/4(beacon)
  Constant1/1 -> MATLAB Function7/6(max_distance_from_ship)
  Memory/1 -> MATLAB Function7/1("stop_flag ")
  MATLAB Function7/2(stop_flag) -> Memory/1, stop_flag/1
  "x,y,psi,r,vc estimate, XY PLANE from x,y,psi"/2(psi_est) -> Sum1/1
  MATLAB Function7/1(yaw_desired) -> Sum1/2
  Sum1/1 -> MATLAB Function/1("angle ")
  MATLAB Function/1(angle) -> angle/1, CONTROLLER1/3(yaw_err), Scope/1
  dynamic + kinematic models1/5(p) -> p/1, "x,y,psi,r,vc estimate, XY PLANE from x,y,psi"/1(p), "z, v_c_z estimate from DEPTH measurement"/1(p)
  "z, v_c_z estimate from DEPTH measurement"/2(state_est) -> Data Store Write1/1
  "z, v_c_z estimate from DEPTH measurement"/3(P_k_k) -> Data Store Write3/1
  "z, v_c_z estimate from DEPTH measurement"/1(z_est) -> Sum/2
  "x,y,psi,r,vc estimate, XY PLANE from x,y,psi"/5(P_knext_knext) -> Data Store Write2/1
  "x,y,psi,r,vc estimate, XY PLANE from x,y,psi"/4(state_est) -> Data Store Write/1
  "x,y,psi,r,vc estimate, XY PLANE from x,y,psi"/6(state_est_ship_fr_plane) -> state_est_plane/1
  "z, v_c_z estimate from DEPTH measurement"/4(state_est_z_ship_fr) -> state_est_z/1

== system simulation_environment_thesis/PHASE 2   ==
  ship_psi_auv : Inport
  ship_p_auv : Inport  Port=2
  ship_p_ds : Inport  Port=3
  Enable : EnablePort
  CONTROLLER : Subsystem
  Constant : Constant  Value=0
  Data Store Read : DataStoreRead  DataStoreName=x_hat_12_xy_4  IndexOptions=Index vector (dialog)  Indices=1  OutputSizes=1
  Data Store Read1 : DataStoreRead  DataStoreName=x_hat_12_z_4  IndexOptions=Index vector (dialog)  Indices=1  OutputSizes=1
  Data Store Read2 : DataStoreRead  DataStoreName=P0_12_xy_4  IndexOptions=Index vector (dialog)  Indices=1  OutputSizes=1
  Data Store Read3 : DataStoreRead  DataStoreName=P0_12_z_4  IndexOptions=Index vector (dialog)  Indices=1  OutputSizes=1
  Data Store Write : DataStoreWrite  DataStoreName=x_hat_23_4  IndexOptions=Index vector (dialog)  Indices=1  OutputSizes=1
  Data Store Write1 : DataStoreWrite  DataStoreName=P0_23_4  IndexOptions=Index vector (dialog)  Indices=1  OutputSizes=1
  MATLAB Function : MATLAB Function  TreatAsAtomicUnit=on
  MATLAB Function1 : MATLAB Function  TreatAsAtomicUnit=on
  MATLAB Function2 : MATLAB Function  TreatAsAtomicUnit=on
  REFERENCE GENERATOR : Subsystem
  Selector1 : Selector  InputPortWidth=4  IndexOptions=Index vector (dialog)  Indices=[1 2 3]  OutputSizes=1
  Selector2 : Selector  InputPortWidth=3  IndexOptions=Index vector (dialog)  Indices=[3]  OutputSizes=1
  Selector3 : Selector  InputPortWidth=3  IndexOptions=Index vector (dialog)  Indices=[1 2]  OutputSizes=1
  Selector4 : Selector  InputPortWidth=8  IndexOptions=Index vector (dialog)  Indices=[1 2 7 8 4 5]  OutputSizes=1
  Selector5 : Selector  InputPortWidth=8  IndexOptions=Index vector (dialog)  Indices=[3 6]  OutputSizes=1
  Sum : Sum  Inputs=|+-
  Sum1 : Sum  Inputs=|+-
  TAU : Scope
  To Workspace : ToWorkspace  VariableName=switchposition12  SampleTime=-1
  dynamic + kinematic models1 : Subsystem
  "p, v_c, psi, r (3D) estimate, from range, v_c, psi, z measurement" : Subsystem
  v error (robot frame) : Scope
  yaw_err : Scope
  z_err : Scope
  s_p_auv : Outport
  s_psi_auv : Outport  Port=2
  stop_flag_2 : Outport  Port=3
  ds_p_auv : Outport  Port=4
  body speed : Outport  Port=5
  state_est_plane : Outport  Port=6
  state_est_z : Outport  Port=7
  angle : Outport  Port=8
  error speed : Outport  Port=9
  z_ err : Outport  Port=10
  wires:
  "p, v_c, psi, r (3D) estimate, from range, v_c, psi, z measurement"/2(psi_est) -> dynamic + kinematic models1/4(yaw_est), Sum1/2
  CONTROLLER/1(tau) -> dynamic + kinematic models1/1(tau), TAU/1
  dynamic + kinematic models1/1(error speed) -> CONTROLLER/1(v_err), error speed/1, v error (robot frame)/1
  ship_psi_auv/1 -> dynamic + kinematic models1/3(last_yaw__from_ship)
  ship_p_ds/1 -> MATLAB Function1/2(ship_p_ds), MATLAB Function/2(ship_p_ds)
  dynamic + kinematic models1/3(p) -> Selector1/1
  MATLAB Function1/1(ship_p_auv) -> s_p_auv/1
  dynamic + kinematic models1/4(yaw) -> s_psi_auv/1, "p, v_c, psi, r (3D) estimate, from range, v_c, psi, z measurement"/3(psi)
  ship_p_auv/1 -> To Workspace/1, MATLAB Function/1(ship_p_auv)
  Sum1/1 -> MATLAB Function2/1("angle ")
  dynamic + kinematic models1/2(v_tot ds) -> "p, v_c, psi, r (3D) estimate, from range, v_c, psi, z measurement"/2(inert_vel)
  Sum/1 -> CONTROLLER/4(z_err), z_ err/1, z_err/1
  Constant/1 -> Sum/1
  MATLAB Function2/1(angle) -> CONTROLLER/2(psi_err), angle/1, yaw_err/1
  "p, v_c, psi, r (3D) estimate, from range, v_c, psi, z measurement"/1(xyz_est) -> Selector2/1, Selector3/1
  Selector2/1 -> Sum/2
  dynamic + kinematic models1/5(body speed) -> body speed/1
  MATLAB Function/1(ds_p_auv) -> dynamic + kinematic models1/5(ds_p_auv)
  Selector1/1 -> ds_p_auv/1, MATLAB Function1/1(ds_p_auv), "p, v_c, psi, r (3D) estimate, from range, v_c, psi, z measurement"/1(p)
  Data Store Read/1 -> "p, v_c, psi, r (3D) estimate, from range, v_c, psi, z measurement"/4(x_0_hat_xy)
  Data Store Read1/1 -> "p, v_c, psi, r (3D) estimate, from range, v_c, psi, z measurement"/5(x_0_hat_z)
  Data Store Read2/1 -> "p, v_c, psi, r (3D) estimate, from range, v_c, psi, z measurement"/6(P0_xy)
  Data Store Read3/1 -> "p, v_c, psi, r (3D) estimate, from range, v_c, psi, z measurement"/7(P0_z)
  "p, v_c, psi, r (3D) estimate, from range, v_c, psi, z measurement"/5(P023) -> Data Store Write1/1
  "p, v_c, psi, r (3D) estimate, from range, v_c, psi, z measurement"/4(state_est) -> Selector4/1, Selector5/1, Data Store Write/1
  Selector4/1 -> state_est_plane/1
  Selector5/1 -> state_est_z/1
  REFERENCE GENERATOR/1(speed) -> dynamic + kinematic models1/2(ref speed), CONTROLLER/3(speed_0)
  Selector3/1 -> REFERENCE GENERATOR/1(p)
  "p, v_c, psi, r (3D) estimate, from range, v_c, psi, z measurement"/3(v_c_xyz) -> REFERENCE GENERATOR/2(v_c)
  REFERENCE GENERATOR/2(yaw_dock) -> Sum1/1
  REFERENCE GENERATOR/3(stop_flag_2) -> stop_flag_2/1

== system simulation_environment_thesis/PHASE 2.21 ==
  note: i know the beacon is my new
      | reference frame, so (0,0,0)
  note: i know the beacon is my new
      | reference frame, so (0,0,0)
  note: true position to plot the data in matlab
  note: physical data to be sent to
      | the range-measurement simulator
  note: ship_psi_auv
  note: ok, trasformazione coerente
  note: ok cominciano esattamente dalla flag
  ship_psi_auv : Inport
  ship_p_auv : Inport  Port=2
  ship_p_ds : Inport  Port=3
  Enable : EnablePort
  CONTROLLER4 : Subsystem
  Constant : Constant  Value=0
  Constant1 : Constant  Value=min_dist_from_ds
  Constant9 : Constant  Value=desired_speed
  Data Store Read : DataStoreRead  DataStoreName=x_hat_12_xy_2  IndexOptions=Index vector (dialog)  Indices=1  OutputSizes=1
  Data Store Read1 : DataStoreRead  DataStoreName=x_hat_12_z_2  IndexOptions=Index vector (dialog)  Indices=1  OutputSizes=1
  Data Store Read2 : DataStoreRead  DataStoreName=P0_12_xy_2  IndexOptions=Index vector (dialog)  Indices=1  OutputSizes=1
  Data Store Read3 : DataStoreRead  DataStoreName=P0_12_z_2  IndexOptions=Index vector (dialog)  Indices=1  OutputSizes=1
  Data Store Write : DataStoreWrite  DataStoreName=x_hat_23_2  IndexOptions=Index vector (dialog)  Indices=1  OutputSizes=1
  Data Store Write1 : DataStoreWrite  DataStoreName=P0_23_2  IndexOptions=Index vector (dialog)  Indices=1  OutputSizes=1
  MATLAB Function : MATLAB Function  TreatAsAtomicUnit=on
  MATLAB Function1 : MATLAB Function  TreatAsAtomicUnit=on
  MATLAB Function2 : MATLAB Function  TreatAsAtomicUnit=on
  MATLAB Function7 : MATLAB Function  TreatAsAtomicUnit=on
  Memory2 : Memory
  Scope{,1..3} (4 alike) : Scope
  Selector1 : Selector  InputPortWidth=4  IndexOptions=Index vector (dialog)  Indices=[1 2 3]  OutputSizes=1
  Selector2 : Selector  InputPortWidth=3  IndexOptions=Index vector (dialog)  Indices=[3]  OutputSizes=1
  Selector3 : Selector  InputPortWidth=3  IndexOptions=Index vector (dialog)  Indices=[1 2]  OutputSizes=1
  Selector4 : Selector  InputPortWidth=8  IndexOptions=Index vector (dialog)  Indices=[1 2 7 8 4 5]  OutputSizes=1
  Selector5 : Selector  InputPortWidth=8  IndexOptions=Index vector (dialog)  Indices=[3 6]  OutputSizes=1
  Sum : Sum  Inputs=|+-
  Sum1 : Sum  Inputs=|+-
  TAU : Scope
  To Workspace : ToWorkspace  VariableName=switchposition12  SampleTime=-1
  dynamic + kinematic models4 : Subsystem
  new origin : Constant  Value=[0; 0]  VectorParams1D=off
  "p, v_c, psi, r (3D) estimate, from RANGE, v_c, PSI measurement" : Subsystem
  v error (robot frame)5 : Scope
  yaw_err3 : Scope
  z_err : Scope
  s_p_auv : Outport
  s_psi_auv : Outport  Port=2
  stop_flag_2 : Outport  Port=3
  ds_p_auv : Outport  Port=4
  body speed : Outport  Port=5
  state_est_plane : Outport  Port=6
  state_est_z : Outport  Port=7
  angle : Outport  Port=8
  error speed : Outport  Port=9
  z_ err : Outport  Port=10
  wires:
  "p, v_c, psi, r (3D) estimate, from RANGE, v_c, PSI measurement"/2(psi_est) -> dynamic + kinematic models4/4(yaw_est), Sum1/2
  CONTROLLER4/1(tau) -> dynamic + kinematic models4/1(tau), TAU/1
  Constant9/1 -> CONTROLLER4/3(speed_0), MATLAB Function7/5(speed), dynamic + kinematic models4/2(ref speed)
  dynamic + kinematic models4/1(error speed) -> CONTROLLER4/1(v_err), error speed/1, v error (robot frame)5/1
  ship_psi_auv/1 -> dynamic + kinematic models4/3(last_yaw__from_ship)
  ship_p_ds/1 -> MATLAB Function1/2(ship_p_ds), MATLAB Function/2(ship_p_ds)
  dynamic + kinematic models4/3(p) -> Selector1/1
  MATLAB Function1/1(ship_p_auv) -> s_p_auv/1
  dynamic + kinematic models4/4(yaw) -> s_psi_auv/1, "p, v_c, psi, r (3D) estimate, from RANGE, v_c, PSI measurement"/3(psi)
  ship_p_auv/1 -> To Workspace/1, MATLAB Function/1(ship_p_auv)
  Sum1/1 -> MATLAB Function2/1("angle ")
  dynamic + kinematic models4/2(v_tot ds) -> "p, v_c, psi, r (3D) estimate, from RANGE, v_c, PSI measurement"/2(inert_vel)
  Sum/1 -> CONTROLLER4/4(z_err), z_ err/1, z_err/1
  Constant/1 -> Sum/1
  new origin/1 -> MATLAB Function7/4(beacon)
  Constant1/1 -> MATLAB Function7/6(threshold)
  "p, v_c, psi, r (3D) estimate, from RANGE, v_c, PSI measurement"/3(v_c_xyz) -> MATLAB Function7/2(v_c)
  MATLAB Function7/1(yaw_desired) -> Scope3/1, Sum1/1
  MATLAB Function7/2(stop_flag) -> Memory2/1, Scope/1, stop_flag_2/1
  MATLAB Function7/3(ds_dist) -> Scope2/1
  MATLAB Function2/1(angle) -> CONTROLLER4/2(psi_err), angle/1, yaw_err3/1
  Memory2/1 -> MATLAB Function7/1("stop_flag ")
  "p, v_c, psi, r (3D) estimate, from RANGE, v_c, PSI measurement"/1(xyz_est) -> Selector2/1, Selector3/1
  Selector2/1 -> Sum/2
  dynamic + kinematic models4/5(body speed) -> body speed/1
  MATLAB Function/1(ds_p_auv) -> dynamic + kinematic models4/5(ds_p_auv), Scope1/1
  Selector1/1 -> ds_p_auv/1, MATLAB Function1/1(ds_p_auv), "p, v_c, psi, r (3D) estimate, from RANGE, v_c, PSI measurement"/1(p)
  Data Store Read/1 -> "p, v_c, psi, r (3D) estimate, from RANGE, v_c, PSI measurement"/4(x_0_hat_xy)
  Data Store Read1/1 -> "p, v_c, psi, r (3D) estimate, from RANGE, v_c, PSI measurement"/5(x_0_hat_z)
  "p, v_c, psi, r (3D) estimate, from RANGE, v_c, PSI measurement"/4(state_est) -> Selector5/1, Selector4/1, Data Store Write/1
  Data Store Read2/1 -> "p, v_c, psi, r (3D) estimate, from RANGE, v_c, PSI measurement"/6(P0_xy)
  Data Store Read3/1 -> "p, v_c, psi, r (3D) estimate, from RANGE, v_c, PSI measurement"/7(P0_z)
  "p, v_c, psi, r (3D) estimate, from RANGE, v_c, PSI measurement"/5(P023) -> Data Store Write1/1
  Selector4/1 -> state_est_plane/1
  Selector5/1 -> state_est_z/1
  Selector3/1 -> MATLAB Function7/3(p)
